simulink model "MCVT_Integration"  (R2020a)
settings: Solver=ode8  StartTime=0.0  StopTime=T_end  FixedStep=dt  RelTol=1e-3  EnableMultiTasking=on
values from neighbouring files (symbols this model uses):
  assumed_power_voltage = 1.25e2    (= 125)    [MCVT_Input_File.m]    (also in MCVT_Run_QuickCheck.m)
  battery_power_toggle = 1    [MCVT_Input_File.m]    (also in MCVT_Run_QuickCheck.m)
  battery_trickle_charge_param = 0.1    [MCVT_Input_File.m]    (also in MCVT_Run_QuickCheck.m)
  battery_trickle_leakage_param = 0.01    [MCVT_Input_File.m]    (also in MCVT_Run_QuickCheck.m)
  damage_case_id = 3    [MCVT_Input_File.m]    (also in MCVT_Run_QuickCheck.m = 1)
  dt = 1/fs    (= 0.001)    [MCVT_Run_QuickCheck.m]    (also in MCVT_Sim_Set.m)
  dt_2 = 1/fs_2    (= 0.001)    [MCVT_Run_QuickCheck.m]    (also in MCVT_Sim_Set.m)
  dt_3 = 1/fs_3    (= 0.001)    [MCVT_Run_QuickCheck.m]    (also in MCVT_Sim_Set.m)
  dt_5 = 1/fs_5    (= 0.005)    [MCVT_Run_QuickCheck.m]    (also in MCVT_Sim_Set.m)
  dt_8 = 1/fs_8    (= 0.05)    [MCVT_Run_QuickCheck.m]    (also in MCVT_Sim_Set.m)
  dt_9 = 1/fs_9    (= 0.001)    [MCVT_Run_QuickCheck.m]    (also in MCVT_Sim_Set.m)
  dust_met_duration = 3    [MCVT_Input_File.m]    (also in MCVT_Run_QuickCheck.m = 2)
  ECLSS_indoor_set_temperature = 298.15    [MCVT_Input_File.m]    (also in MCVT_Run_QuickCheck.m)
  energy_store_max_o = 150    [MCVT_Input_File.m]    (also in MCVT_Run_QuickCheck.m)
  energy_store_o = 120    [MCVT_Input_File.m]    (also in MCVT_Run_QuickCheck.m)
  fs = 1000    [MCVT_Run_QuickCheck.m]    (also in MCVT_Sim_Set.m)
  gain_charge_param = 1    [MCVT_Input_File.m]    (also in MCVT_Run_QuickCheck.m)
  ground_temperature = 373    [MCVT_Habitat_Design_Input_File.m]    (also in MCVT_Run_QuickCheck.m)
  I_max_charge = 800    [MCVT_Input_File.m]    (also in MCVT_Run_QuickCheck.m)
  I_max_discharge = 800    [MCVT_Input_File.m]    (also in MCVT_Run_QuickCheck.m)
  initial_pressure = 1e5    (= 100000)    [MCVT_Input_File.m]    (also in MCVT_Run_QuickCheck.m)
  initial_temperature = 295    [MCVT_Input_File.m]    (also in MCVT_Run_QuickCheck.m)
  interior_leak_rate = 0.00415    [MCVT_Input_File.m]    (also in MCVT_Run_QuickCheck.m)
  launch_dust_accum_start_time = 10    [MCVT_Input_File.m]    (also in MCVT_Run_QuickCheck.m = 2)
  launch_dust_accum_stop_time = 12    [MCVT_Input_File.m]    (also in MCVT_Run_QuickCheck.m = 3)
  meteorite_impact_time = 2    [MCVT_Input_File.m]    (also in MCVT_Run_QuickCheck.m = 1)
  nuclear_power_toggle = 1    [MCVT_Input_File.m]    (also in MCVT_Run_QuickCheck.m)
  print_interval = 30    [MCVT_Input_File.m]
  SOC_lower_thres = 0.5    [MCVT_Input_File.m]    (also in MCVT_Run_QuickCheck.m)
  SOC_upper_thres = 0.9    [MCVT_Input_File.m]    (also in MCVT_Run_QuickCheck.m)
  solar_power_toggle = 1    [MCVT_Input_File.m]    (also in MCVT_Run_QuickCheck.m)
  space_temperature = 4    [MCVT_Habitat_Design_Input_File.m]    (also in MCVT_Run_QuickCheck.m)
  Start_angle = 90    [MCVT_Input_File.m]    (also in MCVT_Run_QuickCheck.m)
undefined symbols (not defined in the file or in its folder): A_flow_restrictor, ACTIVITY_LOOKUP_TABLE, air, Aref, Bmap, Bmap2, Bmap3, comms, DiscIntNeverNeededParam, DmgSPL, dust_parameters, ECLSS_dust_damage_repair_time, ECLSS_heater_capacity, ECLSS_IPS_restrictor, ECLSS_IPS_setpointPressure, ECLSS_paint_damage_repair_time, ECLSS_radiatorLoop_discreteNumber, ECLSS_Vst, emptyActivity, expected_heat_add, FI, habitat, HIEM2SMM, HIEM2STM, HIEMDamageElements, impact_numbering, initial_SPLT_damage_index, input_air, input_Cond, input_CP, input_discretization, input_EV, input_Evap, input_radiatorLoop, K_ECLSS_dust_single, K_ECLSS_paint_single, K_Structure_damage_single, Kref, kW_to_W, meteoroidDustDep, mFRF, ndelays, nominalDustDep, noverlap, nuclear_toggle_gain, output_limits, panel_paint0, Ps0, reference_temperature, RepairTime_ECLSS_factor, Sensor_Location_SMM, SMM_T0, SMM_T1, SMM_T2, SMM_T3, SMM_T4, SMM_T4_0, SMM_T4_1, SMM_T4_2, solar_FDD_K ... (+73)
libraries: neural x350, simulink x39, xpcutilitieslib x7, xpcrtudplib x7, dspstat3 x1
(part 17 of 43)

== system MCVT_Integration/Sys2 - Structural System/Sys2_Structural_System/Fault Detection and Diagnosis (FDD) with Binary Classifer/Pattern Recognition Neural Network/Segment 5/Process Input 1 ==
  x : Inport  PortDimensions=2304
      mask Display: e = 0.06;c1 = [120 230 180]/255;c2 = ([120 230 180]+0)/(256+128);c3 = ([120 230 180]+128)/(256+128);patch([0 1 1 0],[0 0 1 1],c1);patch([0 1 1 1-e 1-e 0+e],[0 0 1 1-e 0+e 0+e],c2);patch([0 0 1 1-e 0+e 0+e],[0 1 1 1-e 1-e 0+e],c3);plot([0 1 1 0 0],[0 0 1 1 0]);
  mapminmax : neural/Processing Functions/mapminmax  xmin=[1.68890768575103907293746136054e-05;0.000633620276889826708477393424346;0.000222998452801713436957978253794;0.000431368362363082689762971977387;0.000531122108222891451713432164894;0.000638875588403607099462211404983;0.000217499080727287904179698130314;0.00033571014520373724078841637386;0.000883650891093521601016291810993;0.000242430646705370646080968111136;0.00067103379408529537347022353444;0.000239521696822448889891560286358;0.000501080236445445572832091407633;0.000863871047297815617196925508381;0.00252423613512151251772608340218;0.000995225802512294871124409745278;0.00295979465853198541944180099961;0.00244310231614083784787427866547;0.00132274183935789319335740721328;0.00373466698135427798907470808842;0.00950533780988847658288687370032;0.00636647321529854124527725289795;0.0199315682329001611639007762733;0.0376500723155389419027905262283;0.0387981912472401130331789431693;0.051196977057845788339207615536;0.0673427787385200288250430844528;0.0638461560593408250507962975462;0.0763955033837577651700811998126;0.0913011910313194230415234642351;0.0989225096209834231508750690409;0.118764661157944012570553127262;0.139485450597127524918761309891;0.143958699830379649453604429254;0.162799723117799000071670434409;0.182081827056906869755081856965;0.185517912969883846940888361132;0.223296507510160252563125027336;0.23766970717488211661816421838;0.261298622115231360396592208417;0.280301220582296950478706776266;0.316789846953607234780747603509;0.327664746429752629097720273421;0.355940125937668383482304079735;0.39394147857307915128188824383;0.424913714623202320996853131874;0.446543164286157368003671308543;0.490592638822427262734748865114;0.529834971745900107364946052257;0.561464023731898298485987197637;0.601807024327945971897690924379;0.628283606400607652631151722744;0.695691585764373399136673015164;0.756342797930647403603643397219;0.812339788947628593795968754421;0.874624576860951630230545106315;0.947383998295910467746239191911;1.02164452969774033341820995702;1.09446950536608045290165591723;1.16203155465659224709895624983;1.28380084782838022050555082387;1.38648663420456830230875766574;1.5013802768430426493040386049;1.61856842473328321752035208192;1.78864471970885752227786724688;1.90715520796815019011205549759;2.13325915077395178442998258106;2.33955693899204231200883441488;2.62831959748604404580873961095;2.86076278912233084028571283852;3.2399858781226065218561416259;3.65799953181899706322610654752;4.14014405124945916725209826836;4.69514337131540138159380148863;5.42775348447508765303837208194;6.31384689628814488315811104258;7.29956181822256322533348793513;8.23530287306289388027380482526;9.47875575764500588604732911335;10.4221473712958321300448005786;9.65968681642839577250470028957;8.73864017739197329603939579101;7.77657035192810663914997348911;6.86135634224585189144818286877;6.24298299549075430547873111209;5.55638437780984428826513976674;5.12661382077715899185932357796;4.7498497555413763393517001532;4.39495561126797174011926472303;4.12372889003797205020873661852;3.8810031218801799290929466224;3.67455585544049601054439335712;3.52685516907613871850912801165;3.36006096470228765582533014822;3.19982144402288293250080641883;3.073222777237116787318882416;2.96565993129154126251023626537;2.87652399291207716558460560918;2.76578742565531277008972210751;2.67215377282841259898304997478;2.60979981260237492435294370807;2.52980734275704266522666330275;2.47706499748467390276118749171;2.4236581159772008220443240134;2.3477469491196223394524622563;2.31312338769640390978565847035;2.27247188497808805252020647458;2.23198200886471997961280067102;2.18001428492902427080935012782;2.15557115759078588013153421343;2.11483666878069254124739018152;2.0916319275767718544045692397;2.05966215258545792821109898796;2.04122470763908614443948863482;1.99670789465804543461047160235;1.99226297921445771876847175008;1.97106215804673357006038258987;1.95277087190773679381550209655;1.93952058069353117097932681645;1.91281311778953067026520784566;1.88598829576529403162510334369 ...(+71555 chars)]  xmax=[0.12835677215459345701376037141;0.0798687539515710309689922041798;0.0893983748853020981917083531698;0.0846542842357467933966930218048;0.0750785359022218684588168002847;0.0751345480889428901649651493244;0.0809876926647683376137010213824;0.0769164104702976525462787549259;0.0885998999564363842740277732446;0.0856334285518826759942001558557;0.089862381879236827297852130414;0.0888766573112028807068796254498;0.0954020351186388171349506137631;0.100844261378977270715573411053;0.118988122987556690923760527312;0.0978947451415716346501127986812;0.120801786502380548160573425776;0.12290321748270041646300398952;0.128954351648643800176685658698;0.149391771615034335551897015648;0.138471386278368191469212433731;0.141694735226063101007909494911;0.145035619560576922726369275551;0.166376355740876252786719646792;0.163704455605106852233987524414;0.183495397787084263407919593192;0.188406318338223272013109976797;0.197970876730221151396094114716;0.210136332734909114794419338068;0.224568691335280068432922462307;0.239507873121703712682162290548;0.273910304701343088051856966558;0.258570811171733028199781756484;0.27676276971864000042700126869;0.308175919472495118967003691068;0.3127278386579430136116286576;0.339524208611780620170605970998;0.35088465663259621463154758203;0.383120028628687270266794939744;0.386713043326682659461823732272;0.423644450379236425785478559192;0.442979758527389078093250418533;0.478091928215765737952835934266;0.50046923000339527121127503051;0.529092103018611203957277666632;0.559533013580128857356044136395;0.595807029340494787028603695944;0.629461049342701084441387138213;0.665500929619561532035731943324;0.727687386222988674688849641825;0.760068197283769730887570403866;0.799065093170577500636397871858;0.861232209752629485066677261784;0.927396668462378448438698796963;0.98706913292975617668645327285;1.05943149736575481512090846081;1.13412657272904060512530577398;1.23535120848071611199259223213;1.31087001896932142663843023911;1.43427232861149089870878015063;1.54010124923447500044915159378;1.67667287851986723801189782534;1.81250060493626974356118353171;1.98706387908374004780398536241;2.17724523492180610517721106589;2.40822978283025213031010025588;2.68971738603180288151861532242;2.99931742989777605501444668334;3.27772143214611499928423654637;3.67490703031174126280689051782;4.25474617299227464428668099572;4.83873355598919374642719049007;5.64549197242066025381745930645;6.6474507029146812087105899991;7.75876234687570587311711278744;9.06817181019995821600332419621;10.5274007163276834830867301207;12.089601027791161413915688172;12.3721342805232659145531215472;12.6041072568861451941302220803;12.1791365614054285515521769412;11.7641676022881220831095561152;10.4604370876135526202688197372;9.33978827647514187049182510236;8.30290866302726193737271387363;7.32231301449135241199428492109;6.58364838598029944449763206649;6.0456052467461232069467769179;5.5470777642736699419856449822;5.07064415573981364104838576168;4.72546223282695265766051306855;4.44460510722251189719145259005;4.21832258518141145486879395321;3.94852473216521593357697383908;3.76880781309648726917771455192;3.59726203684483758493684035784;3.45126880415592918183165238588;3.34278754774571851271502964664;3.19701740561260772111040751042;3.10710293151563909930246154545;3.0052686724789632322085708438;2.92383441833180368618627653632;2.84284964477317947384449325909;2.81217442725417843263358008699;2.73806534145692070936206619081;2.70311581900790143251356312248;2.62418913332421288586715490965;2.60733800642591040386264467088;2.55624067034431945444339362439;2.50256018390131851347746305692;2.46749872276327497644388131448;2.43929066694271234894131339388;2.39294558812769819766685941431;2.37676597886610618814984263736;2.32570989394255045468185016944;2.27566549985466126670985431701;2.22796724042580374103295071109;2.22351186701559333513955607486;2.25735970751233416820014099358;2.27876332734904396559727501881;2.2999374658804798698952254199;2.29180125084331187679254071554;2.30895361245221986123965507431 ...(+70568 chars)]  ymin=-1  ymax=1
  p : Outport  PortDimensions=2304
      mask Display: e = 0.06;c1 = [120 230 180]/255;c2 = ([120 230 180]+0)/(256+128);c3 = ([120 230 180]+128)/(256+128);patch([0 1 1 0],[0 0 1 1],c1);patch([0 1 1 1-e 1-e 0+e],[0 0 1 1-e 0+e 0+e],c2);patch([0 0 1 1-e 0+e 0+e],[0 1 1 1-e 1-e 0+e],c3);plot([0 1 1 0 0],[0 0 1 1 0]);
  wires:
  x/1 -> mapminmax/1
  mapminmax/1 -> p/1
